simulink model "Simulink_Program"  (R2023b)
settings: Solver=FixedStepAuto  StartTime=0.0  StopTime=20  FixedStep=0.001  RelTol=1e-3
PreLoadFcn:
  | % NXP_MBDT_S32K3 callback: DO NOT REMOVE
  | mbd_s32k3.common.nxp.model_callbacks.preLoadFcn();
PostLoadFcn:
  | % NXP_MBDT_S32K3 callback: DO NOT REMOVE
  | mbd_s32k3.common.nxp.model_callbacks.postLoadFcn();
InitFcn:
  | % NXP_MBDT_S32K3 callback: DO NOT REMOVE
  | mbd_s32k3.common.nxp.model_callbacks.initFcn();
undefined symbols (not defined in the file or in its folder): battery_builder_rtmsupport, DiscIntNeverNeededParam, gcbh
libraries: nesl_utility x116, pack_105s34p_structured_with_balancing_lib x105, fl_lib x16, ee_lib x7, simulink x5, BatteryBalancing x1
(part 3 of 8)

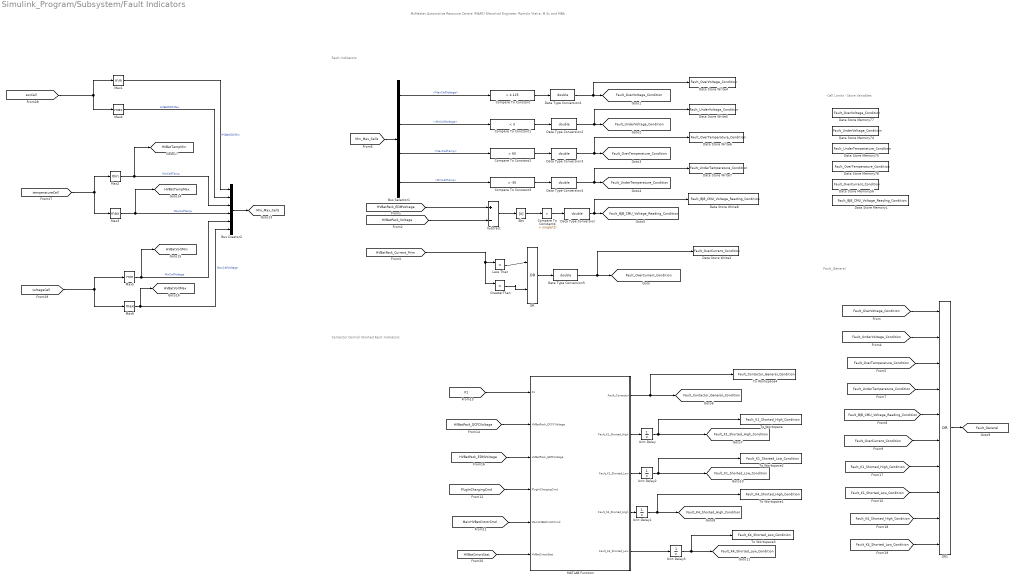
== system Simulink_Program/Subsystem/Fault Indicators ==
  note: Fault indicators
  note: McMaster Automotive Resource Centre (MARC)
      | Electrical Engineer: Romulo Vieira, M.Sc and MBA
  note: Contactor Control Shorted Fault Indicators
  note: Cell Limits - Store Variables
  note: Fault_General
  Abs : Abs  SaturateOnIntegerOverflow=off
  Bus Creator2 : BusCreator  Inputs=6
  Bus Selector1 : BusSelector  OutputSignals=MaxCellVoltage,MinCellVoltage,MaxCellTemp,MinCellTemp
  Compare To Constant : simulink/Logic and Bit Operations/Compare To Constant  relop=>  const=4.125  OutDataTypeStr=boolean
  Compare To Constant1 : simulink/Logic and Bit Operations/Compare To Constant  relop=<  const=3  OutDataTypeStr=boolean
  Compare To Constant2 : simulink/Logic and Bit Operations/Compare To Constant  relop=>  const=60  OutDataTypeStr=boolean
  Compare To Constant3 : simulink/Logic and Bit Operations/Compare To Constant  relop=<  const=-30  OutDataTypeStr=boolean
  Compare To Constant4 : simulink/Logic and Bit Operations/Compare To Constant  relop=>  const=single(2)  OutDataTypeStr=boolean
  Data Store Memory1 : DataStoreMemory  DataStoreName=Fault_BJB_CMU_Voltage_Reading_Condition  ReadBeforeWriteMsg=warning  WriteAfterWriteMsg=warning  WriteAfterReadMsg=warning  ShowAdditionalParam=off  OutDataTypeStr=double
  Data Store Memory56 : DataStoreMemory  DataStoreName=Fault_OverCurrent_Condition  ReadBeforeWriteMsg=warning  WriteAfterWriteMsg=warning  WriteAfterReadMsg=warning  ShowAdditionalParam=off  OutDataTypeStr=double
  Data Store Memory74 : DataStoreMemory  DataStoreName=Fault_UnderVoltage_Condition  ReadBeforeWriteMsg=warning  WriteAfterWriteMsg=warning  WriteAfterReadMsg=warning  ShowAdditionalParam=off  OutDataTypeStr=double
  Data Store Memory75 : DataStoreMemory  DataStoreName=Fault_UnderTemperature_Condition  ReadBeforeWriteMsg=warning  WriteAfterWriteMsg=warning  WriteAfterReadMsg=warning  ShowAdditionalParam=off  OutDataTypeStr=double
  Data Store Memory77 : DataStoreMemory  DataStoreName=Fault_OverVoltage_Condition  ReadBeforeWriteMsg=warning  WriteAfterWriteMsg=warning  WriteAfterReadMsg=warning  ShowAdditionalParam=off  OutDataTypeStr=double
  Data Store Memory78 : DataStoreMemory  DataStoreName=Fault_OverTemperature_Condition  ReadBeforeWriteMsg=warning  WriteAfterWriteMsg=warning  WriteAfterReadMsg=warning  ShowAdditionalParam=off  OutDataTypeStr=double
  Data Store Write2 : DataStoreWrite  DataStoreName=Fault_OverCurrent_Condition  IndexOptions=Index vector (dialog)  Indices=1  OutputSizes=1
  Data Store Write4 : DataStoreWrite  DataStoreName=Fault_OverVoltage_Condition  IndexOptions=Index vector (dialog)  Indices=1  OutputSizes=1
  Data Store Write5 : DataStoreWrite  DataStoreName=Fault_UnderVoltage_Condition  IndexOptions=Index vector (dialog)  Indices=1  OutputSizes=1
  Data Store Write6 : DataStoreWrite  DataStoreName=Fault_OverTemperature_Condition  IndexOptions=Index vector (dialog)  Indices=1  OutputSizes=1
  Data Store Write7 : DataStoreWrite  DataStoreName=Fault_UnderTemperature_Condition  IndexOptions=Index vector (dialog)  Indices=1  OutputSizes=1
  Data Store Write8 : DataStoreWrite  DataStoreName=Fault_BJB_CMU_Voltage_Reading_Condition  IndexOptions=Index vector (dialog)  Indices=1  OutputSizes=1
  Data Type Conversion{,1..5} (6 alike) : DataTypeConversion  OutDataTypeStr=double  SaturateOnIntegerOverflow=off  RndMeth=Floor
  GreaterThan : RelationalOperator  Operator=>  OutDataTypeStr=boolean  RndMeth=Simplest  InputSameDT=off
  Less Than : RelationalOperator  Operator=<  OutDataTypeStr=boolean  RndMeth=Simplest  InputSameDT=off
  MATLAB Function : MATLAB Function  TreatAsAtomicUnit=on
  Max1 : MinMax
  Max2 : MinMax
  Max3 : MinMax  Function=max
  Max4 : MinMax  Function=max
  Max5 : MinMax
  Max6 : MinMax  Function=max
  OR : Logic  Operator=OR  IconShape=distinctive  AllPortsSameDT=off  OutDataTypeStr=boolean
  OR1 : Logic  Operator=OR  Inputs=10  IconShape=distinctive  AllPortsSameDT=off  OutDataTypeStr=boolean
  Subtract : Sum  Inputs=+-
  To Workspace : ToWorkspace  VariableName=Fault_K1_Shorted_High_Condition  SampleTime=-1
  To Workspace1 : ToWorkspace  VariableName=Fault_K4_Shorted_High_Condition  SampleTime=-1
  To Workspace2 : ToWorkspace  VariableName=Fault_K1_Shorted_Low_Condition  SampleTime=-1
  To Workspace3 : ToWorkspace  VariableName=Fault_K4_Shorted_Low_Condition  SampleTime=-1
  To Workspace4 : ToWorkspace  VariableName=Fault_Contactor_General_Condition  SampleTime=-1
  Unit Delay{,1..3} (4 alike) : UnitDelay  SampleTime=-1  HasFrameUpgradeWarning=on
  tag scope: Fault_BJB_CMU_Voltage_Reading_Condition=global, Fault_Contactor_General_Condition=global, Fault_General=global, Fault_K1_Shorted_High_Condition=global, Fault_K1_Shorted_Low_Condition=global, Fault_K4_Shorted_High_Condition=global, Fault_K4_Shorted_Low_Condition=global, Fault_OverCurrent_Condition=global, Fault_OverTemperature_Condition=global, Fault_OverVoltage_Condition=global, Fault_UnderTemperature_Condition=global, Fault_UnderVoltage_Condition=global, HVBatTempMax=global, HVBatTempMin=global, HVBatVoltMax=global, HVBatVoltMin=global, Min_Max_Cells=global
  wires:
  MATLAB Function/1(Fault_Contactor) -> To Workspace4/1, [Fault_Contactor_General_Condition]
  MATLAB Function/2(Fault_K1_Shorted_High) -> Unit Delay/1
  MATLAB Function/4(Fault_K4_Shorted_High) -> Unit Delay1/1
  [Min_Max_Cells] -> Bus Selector1/1
  [HVBatPack_Voltage] -> Subtract/2
  Bus Selector1/2 -> Compare To Constant1/1  "<MinCellVoltage>"
  [HVBatPack_EDMVoltage] -> Subtract/1
  Bus Selector1/1 -> Compare To Constant/1  "<MaxCellVoltage>"
  Compare To Constant2/1 -> Data Type Conversion3/1
  GreaterThan/1 -> OR/2
  Data Type Conversion4/1 -> Data Store Write7/1, [Fault_UnderTemperature_Condition]
  Data Type Conversion3/1 -> Data Store Write6/1, [Fault_OverTemperature_Condition]
  Compare To Constant4/1 -> Data Type Conversion/1
  Data Type Conversion5/1 -> Data Store Write2/1, [Fault_OverCurrent_Condition]
  Less Than/1 -> OR/1
  Compare To Constant3/1 -> Data Type Conversion4/1
  OR/1 -> Data Type Conversion5/1
  Abs/1 -> Compare To Constant4/1
  Data Type Conversion1/1 -> Data Store Write4/1, [Fault_OverVoltage_Condition]
  Data Type Conversion2/1 -> Data Store Write5/1, [Fault_UnderVoltage_Condition]
  Subtract/1 -> Abs/1
  Data Type Conversion/1 -> Data Store Write8/1, [Fault_BJB_CMU_Voltage_Reading_Condition]
  Compare To Constant1/1 -> Data Type Conversion2/1
  [HVBatPack_Current_Prim] -> GreaterThan/1, Less Than/1
  Bus Selector1/3 -> Compare To Constant2/1  "<MaxCellTemp>"
  Compare To Constant/1 -> Data Type Conversion1/1
  Bus Selector1/4 -> Compare To Constant3/1  "<MinCellTemp>"
  [PlugInChargingCmd] -> MATLAB Function/4(PlugInChargingCmd)
  [MainHVBatCntctrCmd] -> MATLAB Function/5(MainHVBatCntctrCmd)
  [HVBatPack_EDMVoltage] -> MATLAB Function/3(HVBatPack_EDMVoltage)
  [HVBatPack_DCFCVoltage] -> MATLAB Function/2(HVBatPack_DCFCVoltage)
  [K1] -> MATLAB Function/1(K1)
  [Fault_OverVoltage_Condition] -> OR1/1
  [Fault_OverCurrent_Condition] -> OR1/6
  [Fault_BJB_CMU_Voltage_Reading_Condition] -> OR1/5
  [Fault_UnderTemperature_Condition] -> OR1/4
  [Fault_OverTemperature_Condition] -> OR1/3
  [Fault_UnderVoltage_Condition] -> OR1/2
  OR1/1 -> [Fault_General]
  [Fault_K1_Shorted_High_Condition] -> OR1/7
  Unit Delay/1 -> To Workspace/1, [Fault_K1_Shorted_High_Condition]
  Unit Delay1/1 -> To Workspace1/1, [Fault_K4_Shorted_High_Condition]
  Unit Delay2/1 -> To Workspace2/1, [Fault_K1_Shorted_Low_Condition]
  MATLAB Function/3(Fault_K1_Shorted_Low) -> Unit Delay2/1
  Unit Delay3/1 -> To Workspace3/1, [Fault_K4_Shorted_Low_Condition]
  MATLAB Function/5(Fault_K4_Shorted_Low) -> Unit Delay3/1
  [Fault_K1_Shorted_Low_Condition] -> OR1/8
  [Fault_K4_Shorted_High_Condition] -> OR1/9
  [Fault_K4_Shorted_Low_Condition] -> OR1/10
  [HVBatCntcrtStat] -> MATLAB Function/6(HVBatCntctrStat)
  Max5/1 -> [HVBatVoltMin], Bus Creator2/5  "MinCellVoltage"
  Max4/1 -> Bus Creator2/2  "HVBatSOCMax"
  Max6/1 -> [HVBatVoltMax], Bus Creator2/6  "MaxCellVoltage"
  Max3/1 -> [HVBatTempMax], Bus Creator2/4  "MaxCellTemp"
  [voltageCell] -> Max5/1, Max6/1
  [temperatureCell] -> Max2/1, Max3/1
  Max2/1 -> [HVBatTempMin], Bus Creator2/3  "MinCellTemp"
  [socCell] -> Max1/1, Max4/1
  Max1/1 -> Bus Creator2/1  "HVBatSOCMin"
  Bus Creator2/1 -> [Min_Max_Cells]

== system Simulink_Program/Subsystem/HVIL ==
  note: McMaster Automotive Resource Centre (MARC)
      | Electrical Engineer: Romulo Vieira, M.Sc and MBA
  HVBatInitHVIL : Constant
  "HVBatInitHVIL   " : Outport
  wires:
  HVBatInitHVIL/1 -> "HVBatInitHVIL   "/1

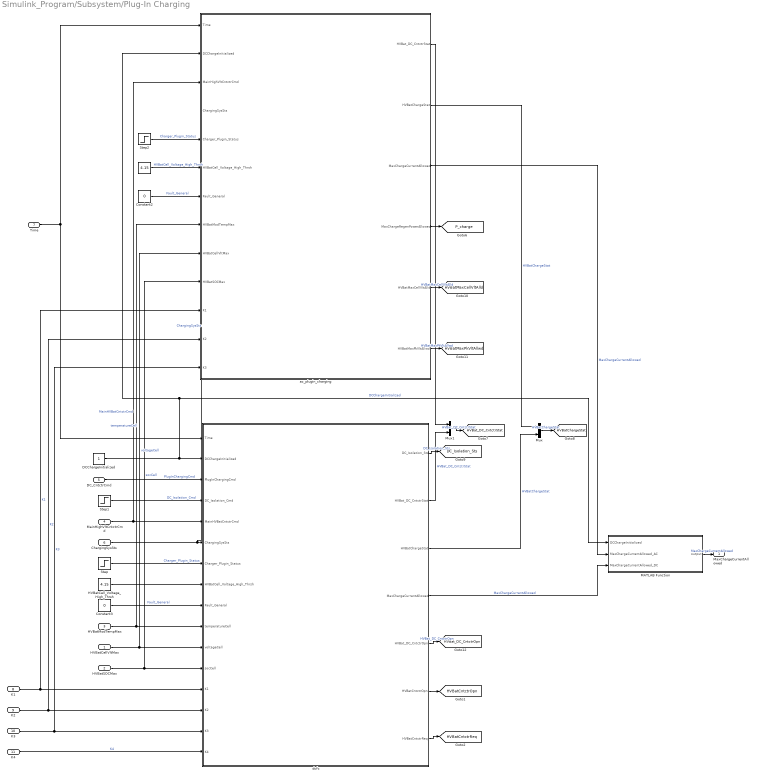
== system Simulink_Program/Subsystem/Plug-In Charging ==
  HVBatCellVltMax : Inport
  HVBatSOCMax : Inport  Port=2
  HVBatModTempMax : Inport  Port=3
  MainHighVltCntctrCmd : Inport  Port=4
  DC_CntctrCmd : Inport  Port=5
  ChargingSysSts : Inport  Port=6
  Time : Inport  Port=7
  K1 : Inport  Port=8
  K2 : Inport  Port=9
  K3 : Inport  Port=10
  K4 : Inport  Port=11
  " " : Constant  Value=4.15
  Constant2 : Constant  Value=0
  Constant3 : Constant  Value=0
  DCChargeInitialized : Constant
  HVBatCell_Voltage_High_Thrsh : Constant  Value=4.15
  MATLAB Function : MATLAB Function  TreatAsAtomicUnit=on
  Mux : Mux  Inputs=2
  Mux1 : Mux  Inputs=2
  Step : Step  Time=0  SampleTime=0.001
  Step1 : Step  Time=0  SampleTime=0.001
  Step2 : Step  Time=0
  ac_plugin_charging : MATLAB Function  TreatAsAtomicUnit=on
  dcfc : MATLAB Function  TreatAsAtomicUnit=on
  MaxChargeCurrentAllowed : Outport
  tag scope: DC_Isolation_Sts=global, HVBatChargeStat=global, HVBatCntctrOpn=global, HVBatCntctrReq=global, HVBatMaxCellVltAlld=global, HVBatMaxPkVltAllwd=global, HVBat_DC_CntctrOpn=global, HVBat_DC_CntctrStat=global, P_charge=global
  wires:
  K4/1 -> dcfc/16(K4)  "K4"
  Step1/1 -> dcfc/4(DC_Isolation_Cmd)  "DC_Isolation_Cmd"
  Time/1 -> dcfc/1(Time), ac_plugin_charging/1(Time)
  Step/1 -> dcfc/7(Charger_Plugin_Status)  "Charger_Plugin_Status"
  dcfc/1(DC_Isolation_Sts) -> [DC_Isolation_Sts]  "DC_Isolation_Sts"
  ac_plugin_charging/5(HVBatMaxCellVltAlld) -> [HVBatMaxCellVltAlld]  "HVBatMaxCellVltAlld"
  ac_plugin_charging/6(HVBatMaxPkVltAllwd) -> [HVBatMaxPkVltAllwd]  "HVBatMaxPkVltAllwd"
  ac_plugin_charging/3(MaxChargeCurrentAllowed) -> MATLAB Function/2(MaxChargeCurrentAllowed_AC)  "MaxChargeCurrentAllowed"
  K1/1 -> dcfc/13(K1), ac_plugin_charging/11(K1)  "K1"
  K2/1 -> dcfc/14(K2), ac_plugin_charging/12(K2)  "K2"
  K3/1 -> dcfc/15(K3), ac_plugin_charging/13(K3)  "K3"
  HVBatCell_Voltage_High_Thrsh/1 -> dcfc/8(HVBatCell_Voltage_High_Thrsh)
  ac_plugin_charging/2(HVBatChargeStat) -> Mux/1  "HVBatChargeStat"
  DCChargeInitialized/1 -> ac_plugin_charging/2(DCChargeInitialized), MATLAB Function/1(DCChargeInitialized), dcfc/2(DCChargeInitialized)  "DCChargeInitialized"
  Mux/1 -> [HVBatChargeStat]  "HVBatChargeStat"
  dcfc/3(HVBatChargeStat) -> Mux/2  "HVBatChargeStat"
  Constant2/1 -> ac_plugin_charging/7(Fault_General)  "Fault_General"
  " "/1 -> ac_plugin_charging/6(HVBatCell_Voltage_High_Thrsh)  "HVBatCell_Voltage_High_Thrsh"
  Step2/1 -> ac_plugin_charging/5(Charger_Plugin_Status)  "Charger_Plugin_Status"
  DC_CntctrCmd/1 -> dcfc/3(PlugInChargingCmd)  "PlugInChargingCmd"
  Constant3/1 -> dcfc/9(Fault_General)  "Fault_General"
  ChargingSysSts/1 -> ac_plugin_charging/4(ChargingSysSts), dcfc/6(ChargingSysSts)  "ChargingSysSts"
  dcfc/4(MaxChargeCurrentAllowed) -> MATLAB Function/3(MaxChargeCurrentAllowed_DC)  "MaxChargeCurrentAllowed"
  MATLAB Function/1(output) -> MaxChargeCurrentAllowed/1  "MaxChargeCurrentAllowed"
  Mux1/1 -> [HVBat_DC_CntctrStat]  "HVBat_DC_CntctrStat"
  dcfc/2(HVBat_DC_CntctrStat) -> Mux1/2  "HVBat_DC_CntctrStat"
  ac_plugin_charging/1(HVBat_DC_CntctrStat) -> Mux1/1
  ac_plugin_charging/4(MaxChargeRegenPowerAllowed) -> [P_charge]
  dcfc/5(HVBat_DC_CntctrOpn) -> [HVBat_DC_CntctrOpn]  "HVBat_DC_CntctrOpn"
  dcfc/6(HVBatCntctrOpn) -> [HVBatCntctrOpn]
  dcfc/7(HVBatCntctrReq) -> [HVBatCntctrReq]
  MainHighVltCntctrCmd/1 -> dcfc/5(MainHVBatCntctrCmd), ac_plugin_charging/3(MainHighVltCntctrCmd)  "MainHVBatCntctrCmd"
  HVBatModTempMax/1 -> dcfc/10(temperatureCell), ac_plugin_charging/8(HVBatModTempMax)  "temperatureCell"
  HVBatCellVltMax/1 -> dcfc/11(voltageCell), ac_plugin_charging/9(HVBatCellVltMax)  "voltageCell"
  HVBatSOCMax/1 -> dcfc/12(socCell), ac_plugin_charging/10(HVBatSOCMax)  "socCell"

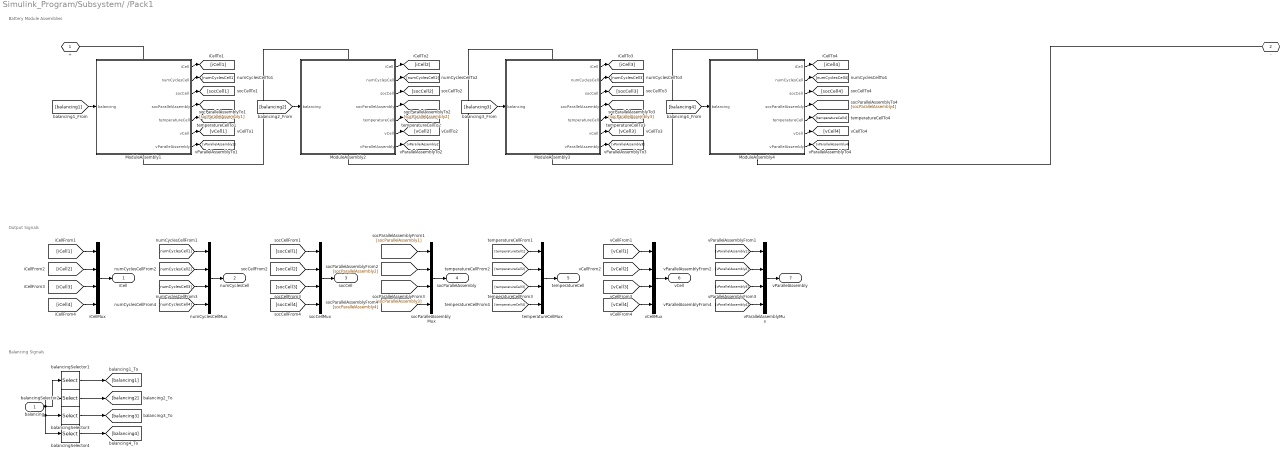
== system Simulink_Program/Subsystem/ /Pack1 ==
  note: Battery Module Assemblies
  note: Output Signals
  note: Balancing Signals
  balancing : Inport  PortDimensions=105
  ModuleAssembly1 : Subsystem  LoadFcn=battery_builder_rtmsupport('loadfcn',gcbh);
      settings: CopyFcn=battery_builder_rtmsupport('copyfcn',gcbh);  PreCopyFcn=battery_builder_rtmsupport('precopyfcn',gcbh);  PreDeleteFcn=battery_builder_rtmsupport('predeletefcn',gcbh);  PortSchema="{\"entries\":[{\"content\":{\"sides\":[{\"content\":{\"connectorIds\":[\"LConn1\"]},\"type\":\"ConnectorPlacement.EquallySpacedRectSide\",\"uuid\":\"5e007e92-2246-4b9f-a838-21fe4b771105\"},{\"content\":{\"connectorIds\":[\"RConn1\"],\"side\":\"RIGHT\"},\"type\":\"ConnectorPlacement.EquallySpacedRectSide\",\"uuid\":\"c53269da-b12c-4944-8bcf-f548990142fd\"},{\"content\":{\"connectorIds\":[\"In1\"],\"side\":\"TOP\"},\"type\":\"ConnectorPlacement.EquallySpacedRectSide\",\"uuid\":\"229c8070-2bae-4d06-bc1c-b3e24a21fda1\"},{\"content\":{\"connectorIds\":[\"Out1\",\"Out2\",\"Out3\",\"Out4\",\"Out5\",\"Out6\",\"Out7\"],\"side\":\"BOTTOM\"},\"type\":\"ConnectorPlacement.EquallySpacedRectSide\",\"uuid\":\"e657053c-c9ef-4453-912e-ffada2428f72\"}]},\"type\":\"ConnectorPlacement.EquallySpacedConnector\",\"uuid\":\"d4cf6711-9d91-4f90-9da8-75b40740f7c2\"}],\"packageUris\":[\"http://schema.mathworks.com/mf0/Connector/R2020b\"],\"version\":\"1.0\"}"
      mask Display: image('$imagefile')
  ModuleAssembly2 : Subsystem  LoadFcn=battery_builder_rtmsupport('loadfcn',gcbh);
      settings: CopyFcn=battery_builder_rtmsupport('copyfcn',gcbh);  PreCopyFcn=battery_builder_rtmsupport('precopyfcn',gcbh);  PreDeleteFcn=battery_builder_rtmsupport('predeletefcn',gcbh);  PortSchema="{\"entries\":[{\"content\":{\"sides\":[{\"content\":{\"connectorIds\":[\"LConn1\"]},\"type\":\"ConnectorPlacement.EquallySpacedRectSide\",\"uuid\":\"5e007e92-2246-4b9f-a838-21fe4b771105\"},{\"content\":{\"connectorIds\":[\"RConn1\"],\"side\":\"RIGHT\"},\"type\":\"ConnectorPlacement.EquallySpacedRectSide\",\"uuid\":\"c53269da-b12c-4944-8bcf-f548990142fd\"},{\"content\":{\"connectorIds\":[\"In1\"],\"side\":\"TOP\"},\"type\":\"ConnectorPlacement.EquallySpacedRectSide\",\"uuid\":\"229c8070-2bae-4d06-bc1c-b3e24a21fda1\"},{\"content\":{\"connectorIds\":[\"Out1\",\"Out2\",\"Out3\",\"Out4\",\"Out5\",\"Out6\",\"Out7\"],\"side\":\"BOTTOM\"},\"type\":\"ConnectorPlacement.EquallySpacedRectSide\",\"uuid\":\"354dc4c9-48a4-4ba8-85e8-851b3319512e\"}]},\"type\":\"ConnectorPlacement.EquallySpacedConnector\",\"uuid\":\"d4cf6711-9d91-4f90-9da8-75b40740f7c2\"}],\"packageUris\":[\"http://schema.mathworks.com/mf0/Connector/R2020b\"],\"version\":\"1.0\"}"
      mask Display: image('$imagefile')
  ModuleAssembly3 : Subsystem  LoadFcn=battery_builder_rtmsupport('loadfcn',gcbh);
      settings: CopyFcn=battery_builder_rtmsupport('copyfcn',gcbh);  PreCopyFcn=battery_builder_rtmsupport('precopyfcn',gcbh);  PreDeleteFcn=battery_builder_rtmsupport('predeletefcn',gcbh);  PortSchema="{\"entries\":[{\"content\":{\"sides\":[{\"content\":{\"connectorIds\":[\"LConn1\"]},\"type\":\"ConnectorPlacement.EquallySpacedRectSide\",\"uuid\":\"5e007e92-2246-4b9f-a838-21fe4b771105\"},{\"content\":{\"connectorIds\":[\"RConn1\"],\"side\":\"RIGHT\"},\"type\":\"ConnectorPlacement.EquallySpacedRectSide\",\"uuid\":\"c53269da-b12c-4944-8bcf-f548990142fd\"},{\"content\":{\"connectorIds\":[\"In1\"],\"side\":\"TOP\"},\"type\":\"ConnectorPlacement.EquallySpacedRectSide\",\"uuid\":\"229c8070-2bae-4d06-bc1c-b3e24a21fda1\"},{\"content\":{\"connectorIds\":[\"Out1\",\"Out2\",\"Out3\",\"Out4\",\"Out5\",\"Out6\",\"Out7\"],\"side\":\"BOTTOM\"},\"type\":\"ConnectorPlacement.EquallySpacedRectSide\",\"uuid\":\"354dc4c9-48a4-4ba8-85e8-851b3319512e\"}]},\"type\":\"ConnectorPlacement.EquallySpacedConnector\",\"uuid\":\"d4cf6711-9d91-4f90-9da8-75b40740f7c2\"}],\"packageUris\":[\"http://schema.mathworks.com/mf0/Connector/R2020b\"],\"version\":\"1.0\"}"
      mask Display: image('$imagefile')
  ModuleAssembly4 : Subsystem  LoadFcn=battery_builder_rtmsupport('loadfcn',gcbh);
      settings: CopyFcn=battery_builder_rtmsupport('copyfcn',gcbh);  PreCopyFcn=battery_builder_rtmsupport('precopyfcn',gcbh);  PreDeleteFcn=battery_builder_rtmsupport('predeletefcn',gcbh);  PortSchema="{\"entries\":[{\"content\":{\"sides\":[{\"content\":{\"connectorIds\":[\"LConn1\"]},\"type\":\"ConnectorPlacement.EquallySpacedRectSide\",\"uuid\":\"5e007e92-2246-4b9f-a838-21fe4b771105\"},{\"content\":{\"connectorIds\":[\"RConn1\"],\"side\":\"RIGHT\"},\"type\":\"ConnectorPlacement.EquallySpacedRectSide\",\"uuid\":\"c53269da-b12c-4944-8bcf-f548990142fd\"},{\"content\":{\"connectorIds\":[\"In1\"],\"side\":\"TOP\"},\"type\":\"ConnectorPlacement.EquallySpacedRectSide\",\"uuid\":\"229c8070-2bae-4d06-bc1c-b3e24a21fda1\"},{\"content\":{\"connectorIds\":[\"Out1\",\"Out2\",\"Out3\",\"Out4\",\"Out5\",\"Out6\",\"Out7\"],\"side\":\"BOTTOM\"},\"type\":\"ConnectorPlacement.EquallySpacedRectSide\",\"uuid\":\"354dc4c9-48a4-4ba8-85e8-851b3319512e\"}]},\"type\":\"ConnectorPlacement.EquallySpacedConnector\",\"uuid\":\"d4cf6711-9d91-4f90-9da8-75b40740f7c2\"}],\"packageUris\":[\"http://schema.mathworks.com/mf0/Connector/R2020b\"],\"version\":\"1.0\"}"
      mask Display: image('$imagefile')
  balancingSelector1 : Selector  InputPortWidth=105  IndexOptions=Index vector (dialog)  Indices="[1   2   3   4   5   6   7   8   9  10  11  12  13  14  15  16  17  18  19  20  21  22  23  24]"  OutputSizes=1
  balancingSelector2 : Selector  InputPortWidth=105  IndexOptions=Index vector (dialog)  Indices="[25  26  27  28  29  30  31  32  33  34  35  36  37  38  39  40  41  42  43  44  45  46  47  48  49  50  51]"  OutputSizes=1
  balancingSelector3 : Selector  InputPortWidth=105  IndexOptions=Index vector (dialog)  Indices="[52  53  54  55  56  57  58  59  60  61  62  63  64  65  66  67  68  69  70  71  72  73  74  75  76  77  78]"  OutputSizes=1
  balancingSelector4 : Selector  InputPortWidth=105  IndexOptions=Index vector (dialog)  Indices="[79   80   81   82   83   84   85   86   87   88   89   90   91   92   93   94   95   96   97   98   99  100  101  102  103  104  105]"  OutputSizes=1
  iCellMux : Mux
  numCyclesCellMux : Mux
  socCellMux : Mux
  socParallelAssemblyMux : Mux
  temperatureCellMux : Mux
  vCellMux : Mux
  vParallelAssemblyMux : Mux
  + : PMIOPort  Side=Left
  - : PMIOPort  Port=2  Side=Right
  iCell : Outport
  numCyclesCell : Outport  Port=2
  socCell : Outport  Port=3
  socParallelAssembly : Outport  Port=4
  temperatureCell : Outport  Port=5
  vCell : Outport  Port=6
  vParallelAssembly : Outport  Port=7
  wires:
  ModuleAssembly1/1(iCell) -> [iCell1]
  iCellMux/1 -> iCell/1
  [iCell1] -> iCellMux/1
  ModuleAssembly1/2(numCyclesCell) -> [numCyclesCell1]
  numCyclesCellMux/1 -> numCyclesCell/1
  [numCyclesCell1] -> numCyclesCellMux/1
  ModuleAssembly1/3(socCell) -> [socCell1]
  socCellMux/1 -> socCell/1
  [socCell1] -> socCellMux/1
  ModuleAssembly1/4(socParallelAssembly) -> [socParallelAssembly1]
  socParallelAssemblyMux/1 -> socParallelAssembly/1
  [socParallelAssembly1] -> socParallelAssemblyMux/1
  ModuleAssembly1/5(temperatureCell) -> [temperatureCell1]
  temperatureCellMux/1 -> temperatureCell/1
  [temperatureCell1] -> temperatureCellMux/1
  ModuleAssembly1/6(vCell) -> [vCell1]
  vCellMux/1 -> vCell/1
  [vCell1] -> vCellMux/1
  ModuleAssembly1/7(vParallelAssembly) -> [vParallelAssembly1]
  vParallelAssemblyMux/1 -> vParallelAssembly/1
  [vParallelAssembly1] -> vParallelAssemblyMux/1
  ModuleAssembly2/1(iCell) -> [iCell2]
  [iCell2] -> iCellMux/2
  ModuleAssembly2/2(numCyclesCell) -> [numCyclesCell2]
  [numCyclesCell2] -> numCyclesCellMux/2
  ModuleAssembly2/3(socCell) -> [socCell2]
  [socCell2] -> socCellMux/2
  ModuleAssembly2/4(socParallelAssembly) -> [socParallelAssembly2]
  [socParallelAssembly2] -> socParallelAssemblyMux/2
  ModuleAssembly2/5(temperatureCell) -> [temperatureCell2]
  [temperatureCell2] -> temperatureCellMux/2
  ModuleAssembly2/6(vCell) -> [vCell2]
  [vCell2] -> vCellMux/2
  ModuleAssembly2/7(vParallelAssembly) -> [vParallelAssembly2]
  [vParallelAssembly2] -> vParallelAssemblyMux/2
  ModuleAssembly3/1(iCell) -> [iCell3]
  [iCell3] -> iCellMux/3
  ModuleAssembly3/2(numCyclesCell) -> [numCyclesCell3]
  [numCyclesCell3] -> numCyclesCellMux/3
  ModuleAssembly3/3(socCell) -> [socCell3]
  [socCell3] -> socCellMux/3
  ModuleAssembly3/4(socParallelAssembly) -> [socParallelAssembly3]
  [socParallelAssembly3] -> socParallelAssemblyMux/3
  ModuleAssembly3/5(temperatureCell) -> [temperatureCell3]
  [temperatureCell3] -> temperatureCellMux/3
  ModuleAssembly3/6(vCell) -> [vCell3]
  [vCell3] -> vCellMux/3
  ModuleAssembly3/7(vParallelAssembly) -> [vParallelAssembly3]
  [vParallelAssembly3] -> vParallelAssemblyMux/3
  ModuleAssembly4/1(iCell) -> [iCell4]
  [iCell4] -> iCellMux/4
  ModuleAssembly4/2(numCyclesCell) -> [numCyclesCell4]
  [numCyclesCell4] -> numCyclesCellMux/4
  ModuleAssembly4/3(socCell) -> [socCell4]
  [socCell4] -> socCellMux/4
  ModuleAssembly4/4(socParallelAssembly) -> [socParallelAssembly4]
  [socParallelAssembly4] -> socParallelAssemblyMux/4
  ModuleAssembly4/5(temperatureCell) -> [temperatureCell4]
  [temperatureCell4] -> temperatureCellMux/4
  ModuleAssembly4/6(vCell) -> [vCell4]
  [vCell4] -> vCellMux/4
  ModuleAssembly4/7(vParallelAssembly) -> [vParallelAssembly4]
  [vParallelAssembly4] -> vParallelAssemblyMux/4
  [balancing1] -> ModuleAssembly1/1(balancing)
  balancing/1 -> balancingSelector3/1, balancingSelector4/1, balancingSelector1/1, balancingSelector2/1
  balancingSelector1/1 -> [balancing1]
  [balancing2] -> ModuleAssembly2/1(balancing)
  balancingSelector2/1 -> [balancing2]
  [balancing3] -> ModuleAssembly3/1(balancing)
  balancingSelector3/1 -> [balancing3]
  [balancing4] -> ModuleAssembly4/1(balancing)
  balancingSelector4/1 -> [balancing4]
  connections:
  ModuleAssembly2/LConn1 -- ModuleAssembly1/RConn1
  ModuleAssembly3/LConn1 -- ModuleAssembly2/RConn1
  ModuleAssembly4/LConn1 -- ModuleAssembly3/RConn1
  ModuleAssembly1/LConn1 -- +/RConn1
  ModuleAssembly4/RConn1 -- -/RConn1
